simulink model "fft_2p_4kc_18i_18o_core"  (R2021a)
settings: Solver=VariableStepDiscrete  StartTime=0.0  StopTime=2^11*3  RelTol=1e-3
undefined symbols (not defined in the file or in its folder): i
libraries: xbsIndex_r4 x48628, casper_library_misc x100, casper_library_delays x10
(part 2 of 338)

== system fft_2p_4kc_18i_18o_core/fft ==
  sync : Inport
  shift : Inport  Port=2
  in00 : Inport  Port=3
  in01 : Inport  Port=4
  in02 : Inport  Port=5
  in03 : Inport  Port=6
  in04 : Inport  Port=7
  in05 : Inport  Port=8
  in06 : Inport  Port=9
  in07 : Inport  Port=10
  in08 : Inport  Port=11
  in09 : Inport  Port=12
  in010 : Inport  Port=13
  in011 : Inport  Port=14
  in012 : Inport  Port=15
  in013 : Inport  Port=16
  in014 : Inport  Port=17
  in015 : Inport  Port=18
  in016 : Inport  Port=19
  in017 : Inport  Port=20
  in018 : Inport  Port=21
  in019 : Inport  Port=22
  in020 : Inport  Port=23
  in021 : Inport  Port=24
  in022 : Inport  Port=25
  in023 : Inport  Port=26
  in024 : Inport  Port=27
  in025 : Inport  Port=28
  in026 : Inport  Port=29
  in027 : Inport  Port=30
  in028 : Inport  Port=31
  in029 : Inport  Port=32
  in030 : Inport  Port=33
  in031 : Inport  Port=34
  fft_biplex : Subsystem  AncestorBlock=casper_library_ffts/fft_biplex  mask(fft_biplex){n_streams=1, n_inputs=16, FFTSize=7, input_bit_width=18, bin_pt_in=17, coeff_bit_width=18, async=off, add_latency=1, mult_latency=2, bram_latency=2, conv_latency=1, quantization=Round  (unbiased: Even Values), overflow=Wrap, coeffs_bit_limit=9, delays_bit_limit=8, coeff_sharing=on, coeff_decimation=on, max_fanout=4, mult_spec=[2 2 2 2 2 2 2], bitgrowth=off, max_bits=19, hardcode_shifts=off, shift_schedule=[1 1 1 1 1 1 1], dsp48_adders=off}
      mask prompts: n_streams "Number simultaneous streams"; n_inputs "Number simultaneous inputs (2*?)"; FFTSize "Size of FFT: (2^? pts)"; input_bit_width "Input bit width"; bin_pt_in "input binary point"; coeff_bit_width "Coefficient Bit Width"; async "Asynchronous operation"; add_latency "Add Latency"; mult_latency "Mult Latency"; bram_latency "BRAM Latency"; conv_latency "Convert latency"; quantization "Quantization Behavior"; overflow "Overflow Behavior"; coeffs_bit_limit "Number bits above which to store stage's coefficients in BRAM (2^? bits)"; delays_bit_limit "Number bits above which to implent stage's delays in BRAM (2^? bits)"; coeff_sharing "BRAM sharing in coeff storage"; coeff_decimation "Store a fraction of coeff factors where useful"; max_fanout "Maximum fanout"; mult_spec "Multiplier impl. (0=core, 1=embedded, 2=behavioural) (left=1st stage, or just one value)"; bitgrowth "Bit growth instead of shift"; max_bits "Maximum bits to grow to"; hardcode_shifts "Hardcode shift schedule"; shift_schedule "Shift schedule"; dsp48_adders "Use DSP48s for adders"
      mask help: FFT internal block.
      mask init:
      | fft_biplex_init(gcb, ...
      |     'n_streams', n_streams, ...
      |     'n_inputs', n_inputs, ...
      |     'FFTSize', FFTSize, ...
      |     'input_bit_width', input_bit_width, ...
      |     'bin_pt_in', bin_pt_in, ...
      |     'coeff_bit_width', coeff_bit_width, ...
      |     'async', async, ...
      |     'add_latency', add_latency, ...
      |     'mult_latency', mult_latency, ...
      |     'bram_latency', bram_latency, ...
      |     'conv_latency', conv_latency, ...
      |     'quantization', quantization, ...
      |     'overflow', overflow, ...
      |     'coeffs_bit_limit', coeffs_bit_limit, ...
      |     'delays_bit_limit', delays_bit_limit, ...
      |     'coeff_sharing', coeff_sharing, ...
      |     'coeff_decimation', coeff_decimation, ...
      |     'max_fanout', max_fanout, ...
      |     'mult_spec', mult_spec, ...
      |     'bitgrowth', bitgrowth, ...
      |     'max_bits', max_bits, ...
      |     'hardcode_shifts', hardcode_shifts, ...
      |     'shift_schedule', shift_schedule, ...
      |     'dsp48_adders', dsp48_adders);
      settings: AttributesFormatString=7 stages
      mask TypeOptions quantization: Truncate | Round  (unbiased: +/- Inf) | Round  (unbiased: Even Values)
      mask TypeOptions overflow: Wrap | Saturate | Error
      mask Callback hardcode_shifts:
      | hardcode_shifts = get_param(gcb, 'hardcode_shifts');
      | set_param_state(gcb, 'shift_schedule', hardcode_shifts);
      mask Callback dsp48_adders: fft_callback_dsp48_adders(gcb);
      mask DialogControl ParameterTabVar0 Prompt: Basic
      mask DialogControl ParameterTabVar01 Prompt: Latency
      mask DialogControl ParameterTabVar012 Prompt: Implementation
  fft_direct : Subsystem  AncestorBlock=casper_library_ffts/fft_direct  mask(fft_direct){n_streams=1, FFTSize=5, input_bit_width=18, bin_pt_in=17, coeff_bit_width=18, map_tail=on, LargerFFTSize=12, StartStage=8, async=off, floating_point=off, float_type=single, exp_width=8, frac_width=24, add_latency=1, mult_latency=2, bram_latency=2, conv_latency=1, add_pipe_latency=0, mult_pipe_latency=0, quantization=Round  (unbiased: Even Values), overflow=Wrap, coeffs_bit_limit=9, coeff_sharing=on, coeff_decimation=on, coeff_generation=on, cal_bits=1, n_bits_rotation=25, max_fanout=4, mult_spec=[2 2 2 2 2], bitgrowth=off, max_bits=19, hardcode_shifts=off, shift_schedule=[1 1 1 1 1], dsp48_adders=off}
      mask prompts: n_streams "Number simultaneous streams"; FFTSize "Size of FFT: (2^? pts)"; input_bit_width "Input Bit Width"; bin_pt_in "Input binary point"; coeff_bit_width "Coefficient Bit Width"; map_tail "Map Tail of Larger FFT"; LargerFFTSize "Size of Larger FFT (2^?)"; StartStage "Start Stage of Map Tail"; async "Asynchronous operation"; floating_point "floating point"; exp_width "exponent width"; frac_width "fraction width"; add_latency "Add Latency"; mult_latency "Mult Latency"; bram_latency "BRAM Latency"; conv_latency "Convert latency"; add_pipe_latency "Add interface pipeline latency"; mult_pipe_latency "Cmult pipeline latency"; quantization "Quantization Behavior"; overflow "Overflow Behavior"; coeffs_bit_limit "Number bits above which to store stage's coefficients in BRAM (2^? bits)"; coeff_sharing "BRAM sharing in coeff storage"; coeff_decimation "Store a fraction of coeff factors where useful"; coeff_generation "Generate coeffs with multipliers where useful"; cal_bits "Number calibration locations when generating coeffs (2^?)"; n_bits_rotation "Feedback rotation vector resolution"; max_fanout "Maximum fanout"; mult_spec "Multiplier impl. (0=core, 1=embedded, 2=behavioural) (left=1st stage, or just one value)"; bitgrowth "Bit growth instead of shifting"; max_bits "Max bits to growth to"; hardcode_shifts "Hardcode shift schedule"; shift_schedule "Shift schedule"; dsp48_adders "DSP48 adders in butterfly"
      mask help:
      | FFT internal block.
      |
      | Implements an N-point FFT as the classic butterfly structure,
      | with N inputs and N outputs.
      mask init:
      | fft_direct_init(gcb, ...
      |     'n_streams', n_streams, ...
      |     'FFTSize', FFTSize, ...
      |     'input_bit_width', input_bit_width, ...
      |     'bin_pt_in', bin_pt_in, ...
      |     'coeff_bit_width', coeff_bit_width, ...
      |     'map_tail', map_tail, ...
      |     'LargerFFTSize', LargerFFTSize, ...
      |     'StartStage', StartStage, ...
      |     'async', async, ...
      |     'add_latency', add_latency, ...
      |     'mult_latency', mult_latency, ...
      |     'bram_latency', bram_latency, ...
      |     'conv_latency', conv_latency, ...
      |     'add_pipe_latency', add_pipe_latency, ...
      |     'mult_pipe_latency', mult_pipe_latency, ...          
      |     'quantization', quantization, ...
      |     'overflow', overflow, ...
      |     'coeffs_bit_limit', coeffs_bit_limit, ...
      |     'coeff_sharing', coeff_sharing, ...
      |     'coeff_decimation', coeff_decimation, ...
      |     'coeff_generation', coeff_generation, ...
      |     'floating_point', floating_point, ...
      |     'float_type', float_type, ...
      |     'exp_width', exp_width, ...
      |     'frac_width', frac_width, ...      
      |     'cal_bits', cal_bits, ...
      |     'n_bits_rotation', n_bits_rotation, ...
      |     'max_fanout', max_fanout, ...
      |     'mult_spec', mult_spec, ...
      |     'bitgrowth', bitgrowth, ...
      |     'max_bits', max_bits, ...
      |     'hardcode_shifts', hardcode_shifts, ...
      |     'shift_schedule', shift_schedule, ...
      |     'dsp48_adders', dsp48_adders);
      settings: AttributesFormatString=|
      | stages [8:12] of 12
      | [18,18]
      | Round  (unbiased: Even Values)
      | Wrap
      mask Callback map_tail:
      | map_tail = get_param(gcb, 'map_tail');
      | set_param_state(gcb, 'LargerFFTSize', map_tail);
      | set_param_state(gcb, 'StartStage', map_tail);
      mask Callback floating_point:
      | onoff = get_param(gcb, 'floating_point');
      | if strcmp(onoff,'on')
      |     enable_fixpt = 'off';
      |     set_param_state(gcb, 'float_type', 'on')
      | 
      |     f_type = get_param(gcb, 'float_type');
      |     if strcmp(f_type,'custom')
      |         set_param_state(gcb, 'exp_width', 'on')
      |         set_param_state(gcb, 'frac_width', 'on')
      |     else
      |         set_param_state(gcb, 'exp_width', 'off')
      |         set_param_state(gcb, 'frac_width', 'off')
      |     end
      | 
      | 
      | else
      |     enable_fixpt = 'on';
      |     set_param_state(gcb, 'float_type', 'off')
      |     set_param_state(gcb, 'exp_width', 'off')
      |     set_param_state(gcb, 'frac_width', 'off')
      | end
      | 
      | set_param_state(gcb, 'input_bit_width', enable_fixpt)
      | set_param_state(gcb, 'bin_pt_in', enable_fixpt)
      | set_param_state(gcb, 'coeff_bit_width', enable_fixpt)
      mask TypeOptions float_type: single | custom
      mask Callback float_type:
      | f_type = get_param(gcb, 'float_type');
      | if strcmp(f_type,'custom')
      |     set_param_state(gcb, 'exp_width', 'on')
      |     set_param_state(gcb, 'frac_width', 'on')
      | else
      |     set_param_state(gcb, 'exp_width', 'off')
      |     set_param_state(gcb, 'frac_width', 'off')
      | end
      mask TypeOptions quantization: Truncate | Round  (unbiased: +/- Inf) | Round  (unbiased: Even Values)
      mask TypeOptions overflow: Wrap | Saturate | Error
      mask Callback hardcode_shifts:
      | hardcode_shifts = get_param(gcb, 'hardcode_shifts');
      | set_param_state(gcb, 'shift_schedule', hardcode_shifts);
      mask Callback dsp48_adders: fft_callback_dsp48_adders(gcb);
      mask DialogControl ParameterTabVar0 Prompt: Basic
      mask DialogControl ParameterTabVar01 Prompt: Latency
      mask DialogControl ParameterTabVar012 Prompt: Implementation
  fft_unscrambler : Subsystem  AncestorBlock=casper_library_ffts/fft_unscrambler  mask(fft_unscrambler){n_streams=1, FFTSize=12, n_inputs=5, n_bits_in=18, bram_latency=2, coeffs_bit_limit=0, async=off, floating_point=off, float_type=single, exp_width=8, frac_width=24}
      mask prompts: n_streams "Number of simultaneous streams"; FFTSize "Size of FFT: (2^?)"; n_inputs "Number of simultaneous inputs: (2^?)"; n_bits_in "Input bit width"; bram_latency "BRAM Latency"; coeffs_bit_limit "Number bits above which to store map coefficients in BRAM (2^? bits)"; async "Asynchronous operation"; floating_point "floating point"; float_type "float type"; exp_width "exponent width"; frac_width "fraction width"
      mask init:
      | fft_unscrambler_init(gcb, ...
      |     'n_streams', n_streams, ...
      |     'FFTSize',FFTSize, ... 
      |     'n_inputs', n_inputs, ...
      |     'n_bits_in', n_bits_in, ...
      |     'floating_point', floating_point, ...
      |     'float_type', float_type, ...
      |     'exp_width', exp_width, ...
      |     'frac_width', frac_width, ...    
      |     'bram_latency', bram_latency, ...
      |     'coeffs_bit_limit', coeffs_bit_limit, ...
      |     'async', async);
      settings: AttributesFormatString=FFTSize=12, n_inputs=5
      mask Callback floating_point:
      | onoff = get_param(gcb, 'floating_point');
      | if strcmp(onoff,'on')
      |     enable_fixpt = 'off';
      |     set_param_state(gcb, 'float_type', 'on')
      | 
      |     f_type = get_param(gcb, 'float_type');
      |     if strcmp(f_type,'custom')
      |         set_param_state(gcb, 'exp_width', 'on')
      |         set_param_state(gcb, 'frac_width', 'on')
      |     else
      |         set_param_state(gcb, 'exp_width', 'off')
      |         set_param_state(gcb, 'frac_width', 'off')
      |     end
      | 
      | 
      | else
      |     enable_fixpt = 'on';
      |     set_param_state(gcb, 'float_type', 'off')
      |     set_param_state(gcb, 'exp_width', 'off')
      |     set_param_state(gcb, 'frac_width', 'off')
      | end
      | 
      | set_param_state(gcb, 'n_bits_in', enable_fixpt)
      mask TypeOptions float_type: single | custom
      mask Callback float_type:
      | f_type = get_param(gcb, 'float_type');
      | if strcmp(f_type,'custom')
      |     set_param_state(gcb, 'exp_width', 'on')
      |     set_param_state(gcb, 'frac_width', 'on')
      | else
      |     set_param_state(gcb, 'exp_width', 'off')
      |     set_param_state(gcb, 'frac_width', 'off')
      | end
  of_or : xbsIndex_r4/Logical  logical_function=OR  inputs=2  en=off  latency=1  precision=Full  arith_type=Unsigned  n_bits=16  bin_pt=0  align_bp=on  dbl_ovrd=off  block_type=logical
  slice : xbsIndex_r4/Slice  nbits=5  boolean_output=off  mode=Lower Bit Location + Width  bit1=0  base1=MSB of Input  bit0=7  base0=LSB of Input  dbl_ovrd=off  block_type=slice
  sync_out : Outport
  out00 : Outport  Port=2
  out01 : Outport  Port=3
  out02 : Outport  Port=4
  out03 : Outport  Port=5
  out04 : Outport  Port=6
  out05 : Outport  Port=7
  out06 : Outport  Port=8
  out07 : Outport  Port=9
  out08 : Outport  Port=10
  out09 : Outport  Port=11
  out010 : Outport  Port=12
  out011 : Outport  Port=13
  out012 : Outport  Port=14
  out013 : Outport  Port=15
  out014 : Outport  Port=16
  out015 : Outport  Port=17
  out016 : Outport  Port=18
  out017 : Outport  Port=19
  out018 : Outport  Port=20
  out019 : Outport  Port=21
  out020 : Outport  Port=22
  out021 : Outport  Port=23
  out022 : Outport  Port=24
  out023 : Outport  Port=25
  out024 : Outport  Port=26
  out025 : Outport  Port=27
  out026 : Outport  Port=28
  out027 : Outport  Port=29
  out028 : Outport  Port=30
  out029 : Outport  Port=31
  out030 : Outport  Port=32
  out031 : Outport  Port=33
  of : Outport  Port=34
  wires:
  sync/1 -> fft_biplex/1(sync)
  shift/1 -> fft_biplex/2(shift), slice/1
  in00/1 -> fft_biplex/3(pol00_in)
  in01/1 -> fft_biplex/4(pol01_in)
  in02/1 -> fft_biplex/5(pol02_in)
  in03/1 -> fft_biplex/6(pol03_in)
  in04/1 -> fft_biplex/7(pol04_in)
  in05/1 -> fft_biplex/8(pol05_in)
  in06/1 -> fft_biplex/9(pol06_in)
  in07/1 -> fft_biplex/10(pol07_in)
  in08/1 -> fft_biplex/11(pol08_in)
  in09/1 -> fft_biplex/12(pol09_in)
  in010/1 -> fft_biplex/13(pol010_in)
  in011/1 -> fft_biplex/14(pol011_in)
  in012/1 -> fft_biplex/15(pol012_in)
  in013/1 -> fft_biplex/16(pol013_in)
  in014/1 -> fft_biplex/17(pol014_in)
  in015/1 -> fft_biplex/18(pol015_in)
  in016/1 -> fft_biplex/19(pol016_in)
  in017/1 -> fft_biplex/20(pol017_in)
  in018/1 -> fft_biplex/21(pol018_in)
  in019/1 -> fft_biplex/22(pol019_in)
  in020/1 -> fft_biplex/23(pol020_in)
  in021/1 -> fft_biplex/24(pol021_in)
  in022/1 -> fft_biplex/25(pol022_in)
  in023/1 -> fft_biplex/26(pol023_in)
  in024/1 -> fft_biplex/27(pol024_in)
  in025/1 -> fft_biplex/28(pol025_in)
  in026/1 -> fft_biplex/29(pol026_in)
  in027/1 -> fft_biplex/30(pol027_in)
  in028/1 -> fft_biplex/31(pol028_in)
  in029/1 -> fft_biplex/32(pol029_in)
  in030/1 -> fft_biplex/33(pol030_in)
  in031/1 -> fft_biplex/34(pol031_in)
  fft_biplex/34(of) -> of_or/2
  slice/1 -> fft_direct/2(shift)
  fft_biplex/1(sync_out) -> fft_direct/1(sync)
  fft_biplex/2(pol00_out) -> fft_direct/3(in00)
  fft_biplex/3(pol01_out) -> fft_direct/4(in01)
  fft_biplex/4(pol02_out) -> fft_direct/5(in02)
  fft_biplex/5(pol03_out) -> fft_direct/6(in03)
  fft_biplex/6(pol04_out) -> fft_direct/7(in04)
  fft_biplex/7(pol05_out) -> fft_direct/8(in05)
  fft_biplex/8(pol06_out) -> fft_direct/9(in06)
  fft_biplex/9(pol07_out) -> fft_direct/10(in07)
  fft_biplex/10(pol08_out) -> fft_direct/11(in08)
  fft_biplex/11(pol09_out) -> fft_direct/12(in09)
  fft_biplex/12(pol010_out) -> fft_direct/13(in010)
  fft_biplex/13(pol011_out) -> fft_direct/14(in011)
  fft_biplex/14(pol012_out) -> fft_direct/15(in012)
  fft_biplex/15(pol013_out) -> fft_direct/16(in013)
  fft_biplex/16(pol014_out) -> fft_direct/17(in014)
  fft_biplex/17(pol015_out) -> fft_direct/18(in015)
  fft_biplex/18(pol016_out) -> fft_direct/19(in016)
  fft_biplex/19(pol017_out) -> fft_direct/20(in017)
  fft_biplex/20(pol018_out) -> fft_direct/21(in018)
  fft_biplex/21(pol019_out) -> fft_direct/22(in019)
  fft_biplex/22(pol020_out) -> fft_direct/23(in020)
  fft_biplex/23(pol021_out) -> fft_direct/24(in021)
  fft_biplex/24(pol022_out) -> fft_direct/25(in022)
  fft_biplex/25(pol023_out) -> fft_direct/26(in023)
  fft_biplex/26(pol024_out) -> fft_direct/27(in024)
  fft_biplex/27(pol025_out) -> fft_direct/28(in025)
  fft_biplex/28(pol026_out) -> fft_direct/29(in026)
  fft_biplex/29(pol027_out) -> fft_direct/30(in027)
  fft_biplex/30(pol028_out) -> fft_direct/31(in028)
  fft_biplex/31(pol029_out) -> fft_direct/32(in029)
  fft_biplex/32(pol030_out) -> fft_direct/33(in030)
  fft_biplex/33(pol031_out) -> fft_direct/34(in031)
  fft_direct/34(of) -> of_or/1
  of_or/1 -> of/1
  fft_direct/1(sync_out) -> fft_unscrambler/1(sync)
  fft_unscrambler/1(sync_out) -> sync_out/1
  fft_direct/2(out00) -> fft_unscrambler/2(in00)
  fft_unscrambler/2(out00) -> out00/1
  fft_direct/3(out01) -> fft_unscrambler/3(in01)
  fft_unscrambler/3(out01) -> out01/1
  fft_direct/4(out02) -> fft_unscrambler/4(in02)
  fft_unscrambler/4(out02) -> out02/1
  fft_direct/5(out03) -> fft_unscrambler/5(in03)
  fft_unscrambler/5(out03) -> out03/1
  fft_direct/6(out04) -> fft_unscrambler/6(in04)
  fft_unscrambler/6(out04) -> out04/1
  fft_direct/7(out05) -> fft_unscrambler/7(in05)
  fft_unscrambler/7(out05) -> out05/1
  fft_direct/8(out06) -> fft_unscrambler/8(in06)
  fft_unscrambler/8(out06) -> out06/1
  fft_direct/9(out07) -> fft_unscrambler/9(in07)
  fft_unscrambler/9(out07) -> out07/1
  fft_direct/10(out08) -> fft_unscrambler/10(in08)
  fft_unscrambler/10(out08) -> out08/1
  fft_direct/11(out09) -> fft_unscrambler/11(in09)
  fft_unscrambler/11(out09) -> out09/1
  fft_direct/12(out010) -> fft_unscrambler/12(in010)
  fft_unscrambler/12(out010) -> out010/1
  fft_direct/13(out011) -> fft_unscrambler/13(in011)
  fft_unscrambler/13(out011) -> out011/1
  fft_direct/14(out012) -> fft_unscrambler/14(in012)
  fft_unscrambler/14(out012) -> out012/1
  fft_direct/15(out013) -> fft_unscrambler/15(in013)
  fft_unscrambler/15(out013) -> out013/1
  fft_direct/16(out014) -> fft_unscrambler/16(in014)
  fft_unscrambler/16(out014) -> out014/1
  fft_direct/17(out015) -> fft_unscrambler/17(in015)
  fft_unscrambler/17(out015) -> out015/1
  fft_direct/18(out016) -> fft_unscrambler/18(in016)
  fft_unscrambler/18(out016) -> out016/1
  fft_direct/19(out017) -> fft_unscrambler/19(in017)
  fft_unscrambler/19(out017) -> out017/1
  fft_direct/20(out018) -> fft_unscrambler/20(in018)
  fft_unscrambler/20(out018) -> out018/1
  fft_direct/21(out019) -> fft_unscrambler/21(in019)
  fft_unscrambler/21(out019) -> out019/1
  fft_direct/22(out020) -> fft_unscrambler/22(in020)
  fft_unscrambler/22(out020) -> out020/1
  fft_direct/23(out021) -> fft_unscrambler/23(in021)
  fft_unscrambler/23(out021) -> out021/1
  fft_direct/24(out022) -> fft_unscrambler/24(in022)
  fft_unscrambler/24(out022) -> out022/1
  fft_direct/25(out023) -> fft_unscrambler/25(in023)
  fft_unscrambler/25(out023) -> out023/1
  fft_direct/26(out024) -> fft_unscrambler/26(in024)
  fft_unscrambler/26(out024) -> out024/1
  fft_direct/27(out025) -> fft_unscrambler/27(in025)
  fft_unscrambler/27(out025) -> out025/1
  fft_direct/28(out026) -> fft_unscrambler/28(in026)
  fft_unscrambler/28(out026) -> out026/1
  fft_direct/29(out027) -> fft_unscrambler/29(in027)
  fft_unscrambler/29(out027) -> out027/1
  fft_direct/30(out028) -> fft_unscrambler/30(in028)
  fft_unscrambler/30(out028) -> out028/1
  fft_direct/31(out029) -> fft_unscrambler/31(in029)
  fft_unscrambler/31(out029) -> out029/1
  fft_direct/32(out030) -> fft_unscrambler/32(in030)
  fft_unscrambler/32(out030) -> out030/1
  fft_direct/33(out031) -> fft_unscrambler/33(in031)
  fft_unscrambler/33(out031) -> out031/1

== system fft_2p_4kc_18i_18o_core/munge ==
  din : Inport
  join : Subsystem  AncestorBlock=casper_library_flow_control/bus_create  mask(bus_create){inputNum=64}
      mask prompts: inputNum "Number of inputs:"
      mask init:
      | bus_create_init(gcb,...
      |     'inputNum', inputNum);
  reinterpret : xbsIndex_r4/Reinterpret  force_arith_type=on  arith_type=Unsigned  force_bin_pt=on  bin_pt=0  block_type=reinterpret
  reinterpret_out : xbsIndex_r4/Reinterpret  force_arith_type=on  arith_type=Unsigned  force_bin_pt=on  bin_pt=0  block_type=reinterpret
  split : Subsystem  AncestorBlock=casper_library_flow_control/bus_expand  mask(bus_expand){mode=divisions of arbitrary size, outputNum=64, outputWidth=[18;18;18;18;18;18;18;18;18;18;18;18;18;18;18;18;18;18;18;18;18;18;18;18;18;18;18;18;18;18;18;18;18;18;18;18;18;18;18;18;18;18;18;18;18;18;18;18;18;18;18;18;18;18;18;18;18;18;18;18;18;18;18;18], outputBinaryPt=[0 0 0 0 0 0 0 0 0 0 0 0 0 0 0 0 0 0 0 0 0 0 0 0 0 0 0 0 0 0 0 0 0 0 0 0 0 0 0 0 0 0 0 0 0 0 0 0 0 0 0 0 0 0 0 0 0 0 0 0 0 0 0 0], outputArithmeticType=[0 0 0 0 0 0 0 0 0 0 0 0 0 0 0 0 0 0 0 0 0 0 0 0 0 0 0 0 0 0 0 0 0 0 0 0 0 0 0 0 0 0 0 0 0 0 0 0 0 0 0 0 0 0 0 0 0 0 0 0 0 0 0 0], show_format=off, outputToWorkspace=off, variablePrefix=out, outputToModelAsWell=on}
      mask prompts: outputNum "Number of outputs:"; outputWidth "Output width:"; outputBinaryPt "Output binary point position:"; outputArithmeticType "Output arithmetic type (ufix=0, fix=1, bool=2):"; show_format "Print format string?"; outputToWorkspace "Output to workspace?"; variablePrefix "Variable name prefix:"; outputToModelAsWell "Output to model as well?"
      mask help:
      | Expands a 'bus' made using a bus_create or Xilinx
      | concat block.
      | The input will be divided into the specified number
      | of outputs with the specified arithmetic types.
      | Divisions are made from most significant bit.
      mask init: same as fft_2p_4kc_18i_18o_core/bus_expand
      settings: AttributesFormatString=64 outputs
      mask TypeOptions mode: divisions of equal size | divisions of arbitrary size
      mask Callback mode: %bus_expand_callback();
      mask Callback outputWidth: %bus_expand_callback();
      mask Callback outputToWorkspace: %bus_expand_callback();
  dout : Outport
  wires:
  reinterpret_out/1 -> dout/1
  din/1 -> reinterpret/1
  reinterpret/1 -> split/1(bus_in)
  join/1(bus_out) -> reinterpret_out/1
  split/1(msb_out64) -> join/1(in1)
  split/33(out32) -> join/2(in2)
  split/2(out63) -> join/3(in3)
  split/34(out31) -> join/4(in4)
  split/3(out62) -> join/5(in5)
  split/35(out30) -> join/6(in6)
  split/4(out61) -> join/7(in7)
  split/36(out29) -> join/8(in8)
  split/5(out60) -> join/9(in9)
  split/37(out28) -> join/10(in10)
  split/6(out59) -> join/11(in11)
  split/38(out27) -> join/12(in12)
  split/7(out58) -> join/13(in13)
  split/39(out26) -> join/14(in14)
  split/8(out57) -> join/15(in15)
  split/40(out25) -> join/16(in16)
  split/9(out56) -> join/17(in17)
  split/41(out24) -> join/18(in18)
  split/10(out55) -> join/19(in19)
  split/42(out23) -> join/20(in20)
  split/11(out54) -> join/21(in21)
  split/43(out22) -> join/22(in22)
  split/12(out53) -> join/23(in23)
  split/44(out21) -> join/24(in24)
  split/13(out52) -> join/25(in25)
  split/45(out20) -> join/26(in26)
  split/14(out51) -> join/27(in27)
  split/46(out19) -> join/28(in28)
  split/15(out50) -> join/29(in29)
  split/47(out18) -> join/30(in30)
  split/16(out49) -> join/31(in31)
  split/48(out17) -> join/32(in32)
  split/17(out48) -> join/33(in33)
  split/49(out16) -> join/34(in34)
  split/18(out47) -> join/35(in35)
  split/50(out15) -> join/36(in36)
  split/19(out46) -> join/37(in37)
  split/51(out14) -> join/38(in38)
  split/20(out45) -> join/39(in39)
  split/52(out13) -> join/40(in40)
  split/21(out44) -> join/41(in41)
  split/53(out12) -> join/42(in42)
  split/22(out43) -> join/43(in43)
  split/54(out11) -> join/44(in44)
  split/23(out42) -> join/45(in45)
  split/55(out10) -> join/46(in46)
  split/24(out41) -> join/47(in47)
  split/56(out9) -> join/48(in48)
  split/25(out40) -> join/49(in49)
  split/57(out8) -> join/50(in50)
  split/26(out39) -> join/51(in51)
  split/58(out7) -> join/52(in52)
  split/27(out38) -> join/53(in53)
  split/59(out6) -> join/54(in54)
  split/28(out37) -> join/55(in55)
  split/60(out5) -> join/56(in56)
  split/29(out36) -> join/57(in57)
  split/61(out4) -> join/58(in58)
  split/30(out35) -> join/59(in59)
  split/62(out3) -> join/60(in60)
  split/31(out34) -> join/61(in61)
  split/63(out2) -> join/62(in62)
  split/32(out33) -> join/63(in63)
  split/64(lsb_out1) -> join/64(in64)

== system fft_2p_4kc_18i_18o_core/fft/fft_biplex ==
  sync : Inport
  shift : Inport  Port=2
  pol00_in : Inport  Port=3
  pol01_in : Inport  Port=4
  pol02_in : Inport  Port=5
  pol03_in : Inport  Port=6
  pol04_in : Inport  Port=7
  pol05_in : Inport  Port=8
  pol06_in : Inport  Port=9
  pol07_in : Inport  Port=10
  pol08_in : Inport  Port=11
  pol09_in : Inport  Port=12
  pol010_in : Inport  Port=13
  pol011_in : Inport  Port=14
  pol012_in : Inport  Port=15
  pol013_in : Inport  Port=16
  pol014_in : Inport  Port=17
  pol015_in : Inport  Port=18
  pol016_in : Inport  Port=19
  pol017_in : Inport  Port=20
  pol018_in : Inport  Port=21
  pol019_in : Inport  Port=22
  pol020_in : Inport  Port=23
  pol021_in : Inport  Port=24
  pol022_in : Inport  Port=25
  pol023_in : Inport  Port=26
  pol024_in : Inport  Port=27
  pol025_in : Inport  Port=28
  pol026_in : Inport  Port=29
  pol027_in : Inport  Port=30
  pol028_in : Inport  Port=31
  pol029_in : Inport  Port=32
  pol030_in : Inport  Port=33
  pol031_in : Inport  Port=34
  biplex_core : Subsystem  AncestorBlock=casper_library_ffts/biplex_core  mask(biplex_core){n_inputs=16, FFTSize=7, input_bit_width=18, bin_pt_in=17, coeff_bit_width=18, floating_point=off, float_type=custom, exp_width=8, frac_width=24, add_latency=1, mult_latency=2, bram_latency=2, conv_latency=1, add_pipe_latency=0, mult_pipe_latency=0, quantization=Round  (unbiased: Even Values), overflow=Wrap, delays_bit_limit=8, coeffs_bit_limit=9, coeff_sharing=on, coeff_decimation=on, max_fanout=4, mult_spec=[2 2 2 2 2 2 2], bitgrowth=off, max_bits=19, hardcode_shifts=off, shift_schedule=[1 1 1 1 1 1 1], dsp48_adders=off, async=off}
      mask prompts: n_inputs "Number inputs (2*?)"; FFTSize "Size of FFT: (2^? pts)"; input_bit_width "Input Bit Width"; bin_pt_in "Input binary point"; coeff_bit_width "Coefficient Bit Width"; floating_point "floating point"; float_type "float type"; exp_width "exponent width"; frac_width "fraction width"; add_latency "Add Latency"; mult_latency "Mult Latency"; bram_latency "BRAM Latency"; conv_latency "Convert latency"; add_pipe_latency "Add Interface Pipeline Latency"; mult_pipe_latency "CMult Pipeline Latency"; quantization "Quantization Behavior"; overflow "Overflow Behavior"; delays_bit_limit "Number bits above which to implent stage's delays in BRAM (2^? bits)"; coeffs_bit_limit "Number bits above which to store stage's coefficients in BRAM (2^? bits)"; coeff_sharing "BRAM sharing in coeff storage"; coeff_decimation "Store a fraction of coeff factors where useful"; max_fanout "Maximum fanout"; mult_spec "Multiplier impl. (0=core, 1=embedded, 2=behavioural) (left=1st stage, or just one value)"; bitgrowth "Bit growth instead of shift"; max_bits "Maximum number of bits"; hardcode_shifts "Hardcode shift schedule"; shift_schedule "Shift schedule"; dsp48_adders "Use DSP48s for adders"; async "Asynchronous operation"
      mask help: FFT internal block.
      mask init:
      | biplex_core_init(gcb, ...
      |     'n_inputs', n_inputs, ...
      |     'FFTSize', FFTSize,...
      |     'input_bit_width', input_bit_width,...
      |     'bin_pt_in', bin_pt_in,...
      |     'coeff_bit_width', coeff_bit_width,...
      |     'floating_point', floating_point, ...
      |     'float_type', float_type, ...
      |     'exp_width', exp_width, ...
      |     'frac_width', frac_width, ...       
      |     'async', async, ...
      |     'add_latency', add_latency,...
      |     'mult_latency', mult_latency,...
      |     'bram_latency', bram_latency,...
      |     'conv_latency', conv_latency, ...
      |     'add_pipe_latency', add_pipe_latency, ...
      |     'mult_pipe_latency', mult_pipe_latency, ...        
      |     'quantization', quantization,...
      |     'overflow', overflow,...
      |     'delays_bit_limit', delays_bit_limit, ...
      |     'coeffs_bit_limit', coeffs_bit_limit, ...
      |     'coeff_sharing', coeff_sharing, ...
      |     'coeff_decimation', coeff_decimation, ...
      |     'max_fanout', max_fanout, ...
      |     'mult_spec', mult_spec, ...
      |     'bitgrowth', bitgrowth, ...
      |     'max_bits', max_bits, ...
      |     'hardcode_shifts', hardcode_shifts, ...
      |     'shift_schedule', shift_schedule, ...
      |     'dsp48_adders', dsp48_adders);
      settings: AttributesFormatString=7 stages
      mask Callback floating_point:
      | onoff = get_param(gcb, 'floating_point');
      | if strcmp(onoff,'on')
      |     enable_fixpt = 'off';
      |     set_param_state(gcb, 'float_type', 'on')
      | 
      |     f_type = get_param(gcb, 'float_type');
      |     if strcmp(f_type,'custom')
      |         set_param_state(gcb, 'exp_width', 'on')
      |         set_param_state(gcb, 'frac_width', 'on')
      |     else
      |         set_param_state(gcb, 'exp_width', 'off')
      |         set_param_state(gcb, 'frac_width', 'off')
      |     end
      | 
      | 
      | else
      |     enable_fixpt = 'on';
      |     set_param_state(gcb, 'float_type', 'off')
      |     set_param_state(gcb, 'exp_width', 'off')
      |     set_param_state(gcb, 'frac_width', 'off')
      | end
      | 
      | set_param_state(gcb, 'input_bit_width', enable_fixpt)
      | set_param_state(gcb, 'bin_pt_in', enable_fixpt)
      | set_param_state(gcb, 'coeff_bit_width', enable_fixpt)
      mask TypeOptions float_type: single | custom
      mask Callback float_type:
      | f_type = get_param(gcb, 'float_type');
      | if strcmp(f_type,'custom')
      |     set_param_state(gcb, 'exp_width', 'on')
      |     set_param_state(gcb, 'frac_width', 'on')
      | else
      |     set_param_state(gcb, 'exp_width', 'off')
      |     set_param_state(gcb, 'frac_width', 'off')
      | end
      mask TypeOptions quantization: Truncate | Round  (unbiased: +/- Inf) | Round  (unbiased: Even Values)
      mask TypeOptions overflow: Wrap | Saturate | Error
      mask Callback hardcode_shifts:
      | hardcode_shifts = get_param(gcb, 'hardcode_shifts');
      | set_param_state(gcb, 'shift_schedule', hardcode_shifts);
      mask Callback dsp48_adders: fft_callback_dsp48_adders(gcb);
      mask DialogControl ParameterTabVar0 Prompt: Basic
      mask DialogControl ParameterTabVar01 Prompt: Latency
      mask DialogControl ParameterTabVar012 Prompt: Implementation
  biplex_cplx_unscrambler : Subsystem  AncestorBlock=casper_library_ffts_internal/biplex_cplx_unscrambler  mask(biplex_cplx_unscrambler){FFTSize=7, bram_latency=2, coeffs_bit_limit=9, async=off}
      mask prompts: FFTSize "Size of FFT: (2^? pnts)"; bram_latency "BRAM Latency"; coeffs_bit_limit "Number bits above which to store stage's coefficients in BRAM (2^? bits)"; async "Asynchronous operation"
      mask init:
      | biplex_cplx_unscrambler_init(gcb, ...
      |     'FFTSize', FFTSize, ...
      |     'bram_latency', bram_latency, ...
      |     'coeffs_bit_limit', coeffs_bit_limit, ...
      |     'async', async);
  even_bussify : Subsystem  AncestorBlock=casper_library_flow_control/bus_create  mask(bus_create){inputNum=16}
      mask prompts: inputNum "Number of inputs:"
      mask init:
      | bus_create_init(gcb,...
      |     'inputNum', inputNum);
  odd_bussify : Subsystem  AncestorBlock=casper_library_flow_control/bus_create  mask(bus_create){inputNum=16}
      mask prompts: inputNum "Number of inputs:"
      mask init:
      | bus_create_init(gcb,...
      |     'inputNum', inputNum);
  pol0_debus : Subsystem  AncestorBlock=casper_library_flow_control/bus_expand  mask(bus_expand){mode=divisions of equal size, outputNum=16, outputWidth=36, outputBinaryPt=0, outputArithmeticType=0, show_format=off, outputToWorkspace=off, variablePrefix=out, outputToModelAsWell=on}
      mask prompts: outputNum "Number of outputs:"; outputWidth "Output width:"; outputBinaryPt "Output binary point position:"; outputArithmeticType "Output arithmetic type (ufix=0, fix=1, bool=2):"; show_format "Print format string?"; outputToWorkspace "Output to workspace?"; variablePrefix "Variable name prefix:"; outputToModelAsWell "Output to model as well?"
      mask help:
      | Expands a 'bus' made using a bus_create or Xilinx
      | concat block.
      | The input will be divided into the specified number
      | of outputs with the specified arithmetic types.
      | Divisions are made from most significant bit.
      mask init: same as fft_2p_4kc_18i_18o_core/bus_expand
      settings: AttributesFormatString=16 outputs
      mask TypeOptions mode: divisions of equal size | divisions of arbitrary size
      mask Callback mode: %bus_expand_callback();
      mask Callback outputWidth: %bus_expand_callback();
      mask Callback outputToWorkspace: %bus_expand_callback();
  pol1_debus : Subsystem  AncestorBlock=casper_library_flow_control/bus_expand  mask(bus_expand){mode=divisions of equal size, outputNum=16, outputWidth=36, outputBinaryPt=0, outputArithmeticType=0, show_format=off, outputToWorkspace=off, variablePrefix=out, outputToModelAsWell=on}
      mask prompts: outputNum "Number of outputs:"; outputWidth "Output width:"; outputBinaryPt "Output binary point position:"; outputArithmeticType "Output arithmetic type (ufix=0, fix=1, bool=2):"; show_format "Print format string?"; outputToWorkspace "Output to workspace?"; variablePrefix "Variable name prefix:"; outputToModelAsWell "Output to model as well?"
      mask help:
      | Expands a 'bus' made using a bus_create or Xilinx
      | concat block.
      | The input will be divided into the specified number
      | of outputs with the specified arithmetic types.
      | Divisions are made from most significant bit.
      mask init: same as fft_2p_4kc_18i_18o_core/bus_expand
      settings: AttributesFormatString=16 outputs
      mask TypeOptions mode: divisions of equal size | divisions of arbitrary size
      mask Callback mode: %bus_expand_callback();
      mask Callback outputWidth: %bus_expand_callback();
      mask Callback outputToWorkspace: %bus_expand_callback();
  sync_out : Outport
  pol00_out : Outport  Port=2
  pol01_out : Outport  Port=3
  pol02_out : Outport  Port=4
  pol03_out : Outport  Port=5
  pol04_out : Outport  Port=6
  pol05_out : Outport  Port=7
  pol06_out : Outport  Port=8
  pol07_out : Outport  Port=9
  pol08_out : Outport  Port=10
  pol09_out : Outport  Port=11
  pol010_out : Outport  Port=12
  pol011_out : Outport  Port=13
  pol012_out : Outport  Port=14
  pol013_out : Outport  Port=15
  pol014_out : Outport  Port=16
  pol015_out : Outport  Port=17
  pol016_out : Outport  Port=18
  pol017_out : Outport  Port=19
  pol018_out : Outport  Port=20
  pol019_out : Outport  Port=21
  pol020_out : Outport  Port=22
  pol021_out : Outport  Port=23
  pol022_out : Outport  Port=24
  pol023_out : Outport  Port=25
  pol024_out : Outport  Port=26
  pol025_out : Outport  Port=27
  pol026_out : Outport  Port=28
  pol027_out : Outport  Port=29
  pol028_out : Outport  Port=30
  pol029_out : Outport  Port=31
  pol030_out : Outport  Port=32
  pol031_out : Outport  Port=33
  of : Outport  Port=34
  wires:
  pol00_in/1 -> even_bussify/1(in1)
  pol0_debus/1(msb_out16) -> pol00_out/1
  pol01_in/1 -> odd_bussify/1(in1)
  pol1_debus/1(msb_out16) -> pol01_out/1
  pol02_in/1 -> even_bussify/2(in2)
  pol0_debus/2(out15) -> pol02_out/1
  pol03_in/1 -> odd_bussify/2(in2)
  pol1_debus/2(out15) -> pol03_out/1
  pol04_in/1 -> even_bussify/3(in3)
  pol0_debus/3(out14) -> pol04_out/1
  pol05_in/1 -> odd_bussify/3(in3)
  pol1_debus/3(out14) -> pol05_out/1
  pol06_in/1 -> even_bussify/4(in4)
  pol0_debus/4(out13) -> pol06_out/1
  pol07_in/1 -> odd_bussify/4(in4)
  pol1_debus/4(out13) -> pol07_out/1
  pol08_in/1 -> even_bussify/5(in5)
  pol0_debus/5(out12) -> pol08_out/1
  pol09_in/1 -> odd_bussify/5(in5)
  pol1_debus/5(out12) -> pol09_out/1
  pol010_in/1 -> even_bussify/6(in6)
  pol0_debus/6(out11) -> pol010_out/1
  pol011_in/1 -> odd_bussify/6(in6)
  pol1_debus/6(out11) -> pol011_out/1
  pol012_in/1 -> even_bussify/7(in7)
  pol0_debus/7(out10) -> pol012_out/1
  pol013_in/1 -> odd_bussify/7(in7)
  pol1_debus/7(out10) -> pol013_out/1
  pol014_in/1 -> even_bussify/8(in8)
  pol0_debus/8(out9) -> pol014_out/1
  pol015_in/1 -> odd_bussify/8(in8)
  pol1_debus/8(out9) -> pol015_out/1
  pol016_in/1 -> even_bussify/9(in9)
  pol0_debus/9(out8) -> pol016_out/1
  pol017_in/1 -> odd_bussify/9(in9)
  pol1_debus/9(out8) -> pol017_out/1
  pol018_in/1 -> even_bussify/10(in10)
  pol0_debus/10(out7) -> pol018_out/1
  pol019_in/1 -> odd_bussify/10(in10)
  pol1_debus/10(out7) -> pol019_out/1
  pol020_in/1 -> even_bussify/11(in11)
  pol0_debus/11(out6) -> pol020_out/1
  pol021_in/1 -> odd_bussify/11(in11)
  pol1_debus/11(out6) -> pol021_out/1
  pol022_in/1 -> even_bussify/12(in12)
  pol0_debus/12(out5) -> pol022_out/1
  pol023_in/1 -> odd_bussify/12(in12)
  pol1_debus/12(out5) -> pol023_out/1
  pol024_in/1 -> even_bussify/13(in13)
  pol0_debus/13(out4) -> pol024_out/1
  pol025_in/1 -> odd_bussify/13(in13)
  pol1_debus/13(out4) -> pol025_out/1
  pol026_in/1 -> even_bussify/14(in14)
  pol0_debus/14(out3) -> pol026_out/1
  pol027_in/1 -> odd_bussify/14(in14)
  pol1_debus/14(out3) -> pol027_out/1
  pol028_in/1 -> even_bussify/15(in15)
  pol0_debus/15(out2) -> pol028_out/1
  pol029_in/1 -> odd_bussify/15(in15)
  pol1_debus/15(out2) -> pol029_out/1
  pol030_in/1 -> even_bussify/16(in16)
  pol0_debus/16(lsb_out1) -> pol030_out/1
  pol031_in/1 -> odd_bussify/16(in16)
  pol1_debus/16(lsb_out1) -> pol031_out/1
  sync/1 -> biplex_core/1(sync)
  shift/1 -> biplex_core/2(shift)
  even_bussify/1(bus_out) -> biplex_core/3(pol1)
  odd_bussify/1(bus_out) -> biplex_core/4(pol2)
  biplex_core/1(sync_out) -> biplex_cplx_unscrambler/3(sync)
  biplex_core/2(out1) -> biplex_cplx_unscrambler/1(even)
  biplex_core/3(out2) -> biplex_cplx_unscrambler/2(odd)
  biplex_core/4(of) -> of/1
  biplex_cplx_unscrambler/3(sync_out) -> sync_out/1
  biplex_cplx_unscrambler/1(pol1) -> pol0_debus/1(bus_in)
  biplex_cplx_unscrambler/2(pol2) -> pol1_debus/1(bus_in)
